simulink model "os_polyphase_synth_4096c"  (R2021a) (continued)
== system os_polyphase_synth_4096c/pfb_fir/pol1_in1_tap44 == (continued)
      | PCIN>>17-(D-A)*B-P-CARRYIN
      | PCIN>>17-(D-A)*BCIN
      | PCIN>>17-(D-A)*BCIN-C
      | PCIN>>17-(D-A)*BCIN-C-CARRYIN
      | PCIN>>17-(D-A)*BCIN-CARRYIN
      | PCIN>>17-(D-A)*BCIN-P
      | PCIN>>17-(D-A)*BCIN-P-CARRYIN
      | PCIN>>17-(D-A)-C
      | PCIN>>17-(D-A)-C-CARRYIN
      | PCIN>>17-(D-A)-CARRYIN
      | PCIN>>17-(D-A)-P
      | PCIN>>17-(D-A)-P-CARRYIN
      | PCIN>>17-(D-ACIN)
      | PCIN>>17-(D-ACIN)*(D-ACIN)
      | PCIN>>17-(D-ACIN)*(D-ACIN)-C
      | PCIN>>17-(D-ACIN)*(D-ACIN)-C-CARRYIN
      | PCIN>>17-(D-ACIN)*(D-ACIN)-CARRYIN
      | PCIN>>17-(D-ACIN)*(D-ACIN)-P
      | PCIN>>17-(D-ACIN)*(D-ACIN)-P-CARRYIN
      | PCIN>>17-(D-ACIN)*B
      | PCIN>>17-(D-ACIN)*B-C
      | PCIN>>17-(D-ACIN)*B-C-CARRYIN
      | PCIN>>17-(D-ACIN)*B-CARRYIN
      | PCIN>>17-(D-ACIN)*B-P
      | PCIN>>17-(D-ACIN)*B-P-CARRYIN
      | PCIN>>17-(D-ACIN)*BCIN
      | PCIN>>17-(D-ACIN)*BCIN-C
      | PCIN>>17-(D-ACIN)*BCIN-C-CARRYIN
      | PCIN>>17-(D-ACIN)*BCIN-CARRYIN
      | PCIN>>17-(D-ACIN)*BCIN-P
      | PCIN>>17-(D-ACIN)*BCIN-P-CARRYIN
      | PCIN>>17-(D-ACIN)-C
      | PCIN>>17-(D-ACIN)-C-CARRYIN
      | PCIN>>17-(D-ACIN)-CARRYIN
      | PCIN>>17-(D-ACIN)-P
      | PCIN>>17-(D-ACIN)-P-CARRYIN
      | PCIN>>17-(P)
      | PCIN>>17-(P+(A+D))
      | PCIN>>17-(P+(A+D)*(A+D))
      | PCIN>>17-(P+(A+D)*(A+D)+CARRYIN)
      | PCIN>>17-(P+(A+D)*B)
      | PCIN>>17-(P+(A+D)*B+CARRYIN)
      | PCIN>>17-(P+(A+D)*BCIN)
      | PCIN>>17-(P+(A+D)*BCIN+CARRYIN)
      | PCIN>>17-(P+(A+D)+CARRYIN)
      | PCIN>>17-(P+(ACIN+D))
      | PCIN>>17-(P+(ACIN+D)*(ACIN+D))
      | PCIN>>17-(P+(ACIN+D)*(ACIN+D)+CARRYIN)
      | PCIN>>17-(P+(ACIN+D)*B)
      | PCIN>>17-(P+(ACIN+D)*B+CARRYIN)
      | PCIN>>17-(P+(ACIN+D)*BCIN)
      | PCIN>>17-(P+(ACIN+D)*BCIN+CARRYIN)
      | PCIN>>17-(P+(ACIN+D)+CARRYIN)
      | PCIN>>17-(P+(D+A))
      | PCIN>>17-(P+(D+A)*(D+A))
      | PCIN>>17-(P+(D+A)*(D+A)+CARRYIN)
      | PCIN>>17-(P+(D+A)*B)
      | PCIN>>17-(P+(D+A)*B+CARRYIN)
      | PCIN>>17-(P+(D+A)*BCIN)
      | PCIN>>17-(P+(D+A)*BCIN+CARRYIN)
      | PCIN>>17-(P+(D+A)+CARRYIN)
      | PCIN>>17-(P+(D+ACIN))
      | PCIN>>17-(P+(D+ACIN)*(D+ACIN))
      | PCIN>>17-(P+(D+ACIN)*(D+ACIN)+CARRYIN)
      | PCIN>>17-(P+(D+ACIN)*B)
      | PCIN>>17-(P+(D+ACIN)*B+CARRYIN)
      | PCIN>>17-(P+(D+ACIN)*BCIN)
      | PCIN>>17-(P+(D+ACIN)*BCIN+CARRYIN)
      | PCIN>>17-(P+(D+ACIN)+CARRYIN)
      | PCIN>>17-(P+(D-A))
      | PCIN>>17-(P+(D-A)*(D-A))
      | PCIN>>17-(P+(D-A)*(D-A)+CARRYIN)
      | PCIN>>17-(P+(D-A)*B)
      | PCIN>>17-(P+(D-A)*B+CARRYIN)
      | PCIN>>17-(P+(D-A)*BCIN)
      | PCIN>>17-(P+(D-A)*BCIN+CARRYIN)
      | PCIN>>17-(P+(D-A)+CARRYIN)
      | PCIN>>17-(P+(D-ACIN))
      | PCIN>>17-(P+(D-ACIN)*(D-ACIN))
      | PCIN>>17-(P+(D-ACIN)*(D-ACIN)+CARRYIN)
      | PCIN>>17-(P+(D-ACIN)*B)
      | PCIN>>17-(P+(D-ACIN)*B+CARRYIN)
      | PCIN>>17-(P+(D-ACIN)*BCIN)
      | PCIN>>17-(P+(D-ACIN)*BCIN+CARRYIN)
      | PCIN>>17-(P+(D-ACIN)+CARRYIN)
      | PCIN>>17-(P+A)
      | PCIN>>17-(P+A*A)
      | PCIN>>17-(P+A*A+CARRYIN)
      | PCIN>>17-(P+A*B)
      | PCIN>>17-(P+A*B+CARRYIN)
      | PCIN>>17-(P+A*BCIN)
      | PCIN>>17-(P+A*BCIN+CARRYIN)
      | PCIN>>17-(P+A+CARRYIN)
      | PCIN>>17-(P+ACIN)
      | PCIN>>17-(P+ACIN*ACIN)
      | PCIN>>17-(P+ACIN*ACIN+CARRYIN)
      | PCIN>>17-(P+ACIN*B)
      | PCIN>>17-(P+ACIN*B+CARRYIN)
      | PCIN>>17-(P+ACIN*BCIN)
      | PCIN>>17-(P+ACIN*BCIN+CARRYIN)
      | PCIN>>17-(P+ACIN+CARRYIN)
      | PCIN>>17-(P+B*(A+D))
      | PCIN>>17-(P+B*(A+D)+CARRYIN)
      | PCIN>>17-(P+B*(ACIN+D))
      | PCIN>>17-(P+B*(ACIN+D)+CARRYIN)
      | PCIN>>17-(P+B*(D+A))
      | PCIN>>17-(P+B*(D+A)+CARRYIN)
      | PCIN>>17-(P+B*(D+ACIN))
      | PCIN>>17-(P+B*(D+ACIN)+CARRYIN)
      | PCIN>>17-(P+B*(D-A))
      | PCIN>>17-(P+B*(D-A)+CARRYIN)
      | PCIN>>17-(P+B*(D-ACIN))
      | PCIN>>17-(P+B*(D-ACIN)+CARRYIN)
      | PCIN>>17-(P+B*A)
      | PCIN>>17-(P+B*A+CARRYIN)
      | PCIN>>17-(P+B*ACIN)
      | PCIN>>17-(P+B*ACIN+CARRYIN)
      | PCIN>>17-(P+B*D)
      | PCIN>>17-(P+B*D+CARRYIN)
      | PCIN>>17-(P+BCIN*(A+D))
      | PCIN>>17-(P+BCIN*(A+D)+CARRYIN)
      | PCIN>>17-(P+BCIN*(ACIN+D))
      | PCIN>>17-(P+BCIN*(ACIN+D)+CARRYIN)
      | PCIN>>17-(P+BCIN*(D+A))
      | PCIN>>17-(P+BCIN*(D+A)+CARRYIN)
      | PCIN>>17-(P+BCIN*(D+ACIN))
      | PCIN>>17-(P+BCIN*(D+ACIN)+CARRYIN)
      | PCIN>>17-(P+BCIN*(D-A))
      | PCIN>>17-(P+BCIN*(D-A)+CARRYIN)
      | PCIN>>17-(P+BCIN*(D-ACIN))
      | PCIN>>17-(P+BCIN*(D-ACIN)+CARRYIN)
      | PCIN>>17-(P+BCIN*A)
      | PCIN>>17-(P+BCIN*A+CARRYIN)
      | PCIN>>17-(P+BCIN*ACIN)
      | PCIN>>17-(P+BCIN*ACIN+CARRYIN)
      | PCIN>>17-(P+BCIN*D)
      | PCIN>>17-(P+BCIN*D+CARRYIN)
      | PCIN>>17-(P+C)
      | PCIN>>17-(P+C+C)
      | PCIN>>17-(P+C+C+CARRYIN)
      | PCIN>>17-(P+C+CARRYIN)
      | PCIN>>17-(P+C+CONCAT)
      | PCIN>>17-(P+C+CONCAT+CARRYIN)
      | PCIN>>17-(P+C+P)
      | PCIN>>17-(P+C+P+CARRYIN)
      | PCIN>>17-(P+CARRYIN)
      | PCIN>>17-(P+CONCAT)
      | PCIN>>17-(P+CONCAT+C)
      | PCIN>>17-(P+CONCAT+C+CARRYIN)
      | PCIN>>17-(P+CONCAT+CARRYIN)
      | PCIN>>17-(P+D)
      | PCIN>>17-(P+D*B)
      | PCIN>>17-(P+D*B+CARRYIN)
      | PCIN>>17-(P+D*BCIN)
      | PCIN>>17-(P+D*BCIN+CARRYIN)
      | PCIN>>17-(P+D*D)
      | PCIN>>17-(P+D*D+CARRYIN)
      | PCIN>>17-(P+D+CARRYIN)
      | PCIN>>17-(P+P)
      | PCIN>>17-(P+P+C)
      | PCIN>>17-(P+P+C+CARRYIN)
      | PCIN>>17-(P+P+CARRYIN)
      | PCIN>>17-A
      | PCIN>>17-A*A
      | PCIN>>17-A*A-C
      | PCIN>>17-A*A-C-CARRYIN
      | PCIN>>17-A*A-CARRYIN
      | PCIN>>17-A*A-P
      | PCIN>>17-A*A-P-CARRYIN
      | PCIN>>17-A*B
      | PCIN>>17-A*B-C
      | PCIN>>17-A*B-C-CARRYIN
      | PCIN>>17-A*B-CARRYIN
      | PCIN>>17-A*B-P
      | PCIN>>17-A*B-P-CARRYIN
      | PCIN>>17-A*BCIN
      | PCIN>>17-A*BCIN-C
      | PCIN>>17-A*BCIN-C-CARRYIN
      | PCIN>>17-A*BCIN-CARRYIN
      | PCIN>>17-A*BCIN-P
      | PCIN>>17-A*BCIN-P-CARRYIN
      | PCIN>>17-A-C
      | PCIN>>17-A-C-CARRYIN
      | PCIN>>17-A-CARRYIN
      | PCIN>>17-A-P
      | PCIN>>17-A-P-CARRYIN
      | PCIN>>17-ACIN
      | PCIN>>17-ACIN*ACIN
      | PCIN>>17-ACIN*ACIN-C
      | PCIN>>17-ACIN*ACIN-C-CARRYIN
      | PCIN>>17-ACIN*ACIN-CARRYIN
      | PCIN>>17-ACIN*ACIN-P
      | PCIN>>17-ACIN*ACIN-P-CARRYIN
      | PCIN>>17-ACIN*B
      | PCIN>>17-ACIN*B-C
      | PCIN>>17-ACIN*B-C-CARRYIN
      | PCIN>>17-ACIN*B-CARRYIN
      | PCIN>>17-ACIN*B-P
      | PCIN>>17-ACIN*B-P-CARRYIN
      | PCIN>>17-ACIN*BCIN
      | PCIN>>17-ACIN*BCIN-C
      | PCIN>>17-ACIN*BCIN-C-CARRYIN
      | PCIN>>17-ACIN*BCIN-CARRYIN
      | PCIN>>17-ACIN*BCIN-P
      | PCIN>>17-ACIN*BCIN-P-CARRYIN
      | PCIN>>17-ACIN-C
      | PCIN>>17-ACIN-C-CARRYIN
      | PCIN>>17-ACIN-CARRYIN
      | PCIN>>17-ACIN-P
      | PCIN>>17-ACIN-P-CARRYIN
      | PCIN>>17-B*(A+D)
      | PCIN>>17-B*(A+D)-C
      | PCIN>>17-B*(A+D)-C-CARRYIN
      | PCIN>>17-B*(A+D)-CARRYIN
      | PCIN>>17-B*(A+D)-P
      | PCIN>>17-B*(A+D)-P-CARRYIN
      | PCIN>>17-B*(ACIN+D)
      | PCIN>>17-B*(ACIN+D)-C
      | PCIN>>17-B*(ACIN+D)-C-CARRYIN
      | PCIN>>17-B*(ACIN+D)-CARRYIN
      | PCIN>>17-B*(ACIN+D)-P
      | PCIN>>17-B*(ACIN+D)-P-CARRYIN
      | PCIN>>17-B*(D+A)
      | PCIN>>17-B*(D+A)-C
      | PCIN>>17-B*(D+A)-C-CARRYIN
      | PCIN>>17-B*(D+A)-CARRYIN
      | PCIN>>17-B*(D+A)-P
      | PCIN>>17-B*(D+A)-P-CARRYIN
      | PCIN>>17-B*(D+ACIN)
      | PCIN>>17-B*(D+ACIN)-C
      | PCIN>>17-B*(D+ACIN)-C-CARRYIN
      | PCIN>>17-B*(D+ACIN)-CARRYIN
      | PCIN>>17-B*(D+ACIN)-P
      | PCIN>>17-B*(D+ACIN)-P-CARRYIN
      | PCIN>>17-B*(D-A)
      | PCIN>>17-B*(D-A)-C
      | PCIN>>17-B*(D-A)-C-CARRYIN
      | PCIN>>17-B*(D-A)-CARRYIN
      | PCIN>>17-B*(D-A)-P
      | PCIN>>17-B*(D-A)-P-CARRYIN
      | PCIN>>17-B*(D-ACIN)
      | PCIN>>17-B*(D-ACIN)-C
      | PCIN>>17-B*(D-ACIN)-C-CARRYIN
      | PCIN>>17-B*(D-ACIN)-CARRYIN
      | PCIN>>17-B*(D-ACIN)-P
      | PCIN>>17-B*(D-ACIN)-P-CARRYIN
      | PCIN>>17-B*A
      | PCIN>>17-B*A-C
      | PCIN>>17-B*A-C-CARRYIN
      | PCIN>>17-B*A-CARRYIN
      | PCIN>>17-B*A-P
      | PCIN>>17-B*A-P-CARRYIN
      | PCIN>>17-B*ACIN
      | PCIN>>17-B*ACIN-C
      | PCIN>>17-B*ACIN-C-CARRYIN
      | PCIN>>17-B*ACIN-CARRYIN
      | PCIN>>17-B*ACIN-P
      | PCIN>>17-B*ACIN-P-CARRYIN
      | PCIN>>17-B*D
      | PCIN>>17-B*D-C
      | PCIN>>17-B*D-C-CARRYIN
      | PCIN>>17-B*D-CARRYIN
      | PCIN>>17-B*D-P
      | PCIN>>17-B*D-P-CARRYIN
      | PCIN>>17-BCIN*(A+D)
      | PCIN>>17-BCIN*(A+D)-C
      | PCIN>>17-BCIN*(A+D)-C-CARRYIN
      | PCIN>>17-BCIN*(A+D)-CARRYIN
      | PCIN>>17-BCIN*(A+D)-P
      | PCIN>>17-BCIN*(A+D)-P-CARRYIN
      | PCIN>>17-BCIN*(ACIN+D)
      | PCIN>>17-BCIN*(ACIN+D)-C
      | PCIN>>17-BCIN*(ACIN+D)-C-CARRYIN
      | PCIN>>17-BCIN*(ACIN+D)-CARRYIN
      | PCIN>>17-BCIN*(ACIN+D)-P
      | PCIN>>17-BCIN*(ACIN+D)-P-CARRYIN
      | PCIN>>17-BCIN*(D+A)
      | PCIN>>17-BCIN*(D+A)-C
      | PCIN>>17-BCIN*(D+A)-C-CARRYIN
      | PCIN>>17-BCIN*(D+A)-CARRYIN
      | PCIN>>17-BCIN*(D+A)-P
      | PCIN>>17-BCIN*(D+A)-P-CARRYIN
      | PCIN>>17-BCIN*(D+ACIN)
      | PCIN>>17-BCIN*(D+ACIN)-C
      | PCIN>>17-BCIN*(D+ACIN)-C-CARRYIN
      | PCIN>>17-BCIN*(D+ACIN)-CARRYIN
      | PCIN>>17-BCIN*(D+ACIN)-P
      | PCIN>>17-BCIN*(D+ACIN)-P-CARRYIN
      | PCIN>>17-BCIN*(D-A)
      | PCIN>>17-BCIN*(D-A)-C
      | PCIN>>17-BCIN*(D-A)-C-CARRYIN
      | PCIN>>17-BCIN*(D-A)-CARRYIN
      | PCIN>>17-BCIN*(D-A)-P
      | PCIN>>17-BCIN*(D-A)-P-CARRYIN
      | PCIN>>17-BCIN*(D-ACIN)
      | PCIN>>17-BCIN*(D-ACIN)-C
      | PCIN>>17-BCIN*(D-ACIN)-C-CARRYIN
      | PCIN>>17-BCIN*(D-ACIN)-CARRYIN
      | PCIN>>17-BCIN*(D-ACIN)-P
      | PCIN>>17-BCIN*(D-ACIN)-P-CARRYIN
      | PCIN>>17-BCIN*A
      | PCIN>>17-BCIN*A-C
      | PCIN>>17-BCIN*A-C-CARRYIN
      | PCIN>>17-BCIN*A-CARRYIN
      | PCIN>>17-BCIN*A-P
      | PCIN>>17-BCIN*A-P-CARRYIN
      | PCIN>>17-BCIN*ACIN
      | PCIN>>17-BCIN*ACIN-C
      | PCIN>>17-BCIN*ACIN-C-CARRYIN
      | PCIN>>17-BCIN*ACIN-CARRYIN
      | PCIN>>17-BCIN*ACIN-P
      | PCIN>>17-BCIN*ACIN-P-CARRYIN
      | PCIN>>17-BCIN*D
      | PCIN>>17-BCIN*D-C
      | PCIN>>17-BCIN*D-C-CARRYIN
      | PCIN>>17-BCIN*D-CARRYIN
      | PCIN>>17-BCIN*D-P
      | PCIN>>17-BCIN*D-P-CARRYIN
      | PCIN>>17-C
      | PCIN>>17-C-(A+D)
      | PCIN>>17-C-(A+D)*(A+D)
      | PCIN>>17-C-(A+D)*(A+D)-CARRYIN
      | PCIN>>17-C-(A+D)*B
      | PCIN>>17-C-(A+D)*B-CARRYIN
      | PCIN>>17-C-(A+D)*BCIN
      | PCIN>>17-C-(A+D)*BCIN-CARRYIN
      | PCIN>>17-C-(A+D)-CARRYIN
      | PCIN>>17-C-(ACIN+D)
      | PCIN>>17-C-(ACIN+D)*(ACIN+D)
      | PCIN>>17-C-(ACIN+D)*(ACIN+D)-CARRYIN
      | PCIN>>17-C-(ACIN+D)*B
      | PCIN>>17-C-(ACIN+D)*B-CARRYIN
      | PCIN>>17-C-(ACIN+D)*BCIN
      | PCIN>>17-C-(ACIN+D)*BCIN-CARRYIN
      | PCIN>>17-C-(ACIN+D)-CARRYIN
      | PCIN>>17-C-(D+A)
      | PCIN>>17-C-(D+A)*(D+A)
      | PCIN>>17-C-(D+A)*(D+A)-CARRYIN
      | PCIN>>17-C-(D+A)*B
      | PCIN>>17-C-(D+A)*B-CARRYIN
      | PCIN>>17-C-(D+A)*BCIN
      | PCIN>>17-C-(D+A)*BCIN-CARRYIN
      | PCIN>>17-C-(D+A)-CARRYIN
      | PCIN>>17-C-(D+ACIN)
      | PCIN>>17-C-(D+ACIN)*(D+ACIN)
      | PCIN>>17-C-(D+ACIN)*(D+ACIN)-CARRYIN
      | PCIN>>17-C-(D+ACIN)*B
      | PCIN>>17-C-(D+ACIN)*B-CARRYIN
      | PCIN>>17-C-(D+ACIN)*BCIN
      | PCIN>>17-C-(D+ACIN)*BCIN-CARRYIN
      | PCIN>>17-C-(D+ACIN)-CARRYIN
      | PCIN>>17-C-(D-A)
      | PCIN>>17-C-(D-A)*(D-A)
      | PCIN>>17-C-(D-A)*(D-A)-CARRYIN
      | PCIN>>17-C-(D-A)*B
      | PCIN>>17-C-(D-A)*B-CARRYIN
      | PCIN>>17-C-(D-A)*BCIN
      | PCIN>>17-C-(D-A)*BCIN-CARRYIN
      | PCIN>>17-C-(D-A)-CARRYIN
      | PCIN>>17-C-(D-ACIN)
      | PCIN>>17-C-(D-ACIN)*(D-ACIN)
      | PCIN>>17-C-(D-ACIN)*(D-ACIN)-CARRYIN
      | PCIN>>17-C-(D-ACIN)*B
      | PCIN>>17-C-(D-ACIN)*B-CARRYIN
      | PCIN>>17-C-(D-ACIN)*BCIN
      | PCIN>>17-C-(D-ACIN)*BCIN-CARRYIN
      | PCIN>>17-C-(D-ACIN)-CARRYIN
      | PCIN>>17-C-A
      | PCIN>>17-C-A*A
      | PCIN>>17-C-A*A-CARRYIN
      | PCIN>>17-C-A*B
      | PCIN>>17-C-A*B-CARRYIN
      | PCIN>>17-C-A*BCIN
      | PCIN>>17-C-A*BCIN-CARRYIN
      | PCIN>>17-C-A-CARRYIN
      | PCIN>>17-C-ACIN
      | PCIN>>17-C-ACIN*ACIN
      | PCIN>>17-C-ACIN*ACIN-CARRYIN
      | PCIN>>17-C-ACIN*B
      | PCIN>>17-C-ACIN*B-CARRYIN
      | PCIN>>17-C-ACIN*BCIN
      | PCIN>>17-C-ACIN*BCIN-CARRYIN
      | PCIN>>17-C-ACIN-CARRYIN
      | PCIN>>17-C-B*(A+D)
      | PCIN>>17-C-B*(A+D)-CARRYIN
      | PCIN>>17-C-B*(ACIN+D)
      | PCIN>>17-C-B*(ACIN+D)-CARRYIN
      | PCIN>>17-C-B*(D+A)
      | PCIN>>17-C-B*(D+A)-CARRYIN
      | PCIN>>17-C-B*(D+ACIN)
      | PCIN>>17-C-B*(D+ACIN)-CARRYIN
      | PCIN>>17-C-B*(D-A)
      | PCIN>>17-C-B*(D-A)-CARRYIN
      | PCIN>>17-C-B*(D-ACIN)
      | PCIN>>17-C-B*(D-ACIN)-CARRYIN
      | PCIN>>17-C-B*A
      | PCIN>>17-C-B*A-CARRYIN
      | PCIN>>17-C-B*ACIN
      | PCIN>>17-C-B*ACIN-CARRYIN
      | PCIN>>17-C-B*D
      | PCIN>>17-C-B*D-CARRYIN
      | PCIN>>17-C-BCIN*(A+D)
      | PCIN>>17-C-BCIN*(A+D)-CARRYIN
      | PCIN>>17-C-BCIN*(ACIN+D)
      | PCIN>>17-C-BCIN*(ACIN+D)-CARRYIN
      | PCIN>>17-C-BCIN*(D+A)
      | PCIN>>17-C-BCIN*(D+A)-CARRYIN
      | PCIN>>17-C-BCIN*(D+ACIN)
      | PCIN>>17-C-BCIN*(D+ACIN)-CARRYIN
      | PCIN>>17-C-BCIN*(D-A)
      | PCIN>>17-C-BCIN*(D-A)-CARRYIN
      | PCIN>>17-C-BCIN*(D-ACIN)
      | PCIN>>17-C-BCIN*(D-ACIN)-CARRYIN
      | PCIN>>17-C-BCIN*A
      | PCIN>>17-C-BCIN*A-CARRYIN
      | PCIN>>17-C-BCIN*ACIN
      | PCIN>>17-C-BCIN*ACIN-CARRYIN
      | PCIN>>17-C-BCIN*D
      | PCIN>>17-C-BCIN*D-CARRYIN
      | PCIN>>17-C-C
      | PCIN>>17-C-C-CARRYIN
      | PCIN>>17-C-C-CONCAT
      | PCIN>>17-C-C-CONCAT-CARRYIN
      | PCIN>>17-C-C-P
      | PCIN>>17-C-C-P-CARRYIN
      | PCIN>>17-C-CARRYIN
      | PCIN>>17-C-CONCAT
      | PCIN>>17-C-CONCAT-C
      | PCIN>>17-C-CONCAT-C-CARRYIN
      | PCIN>>17-C-CONCAT-CARRYIN
      | PCIN>>17-C-CONCAT-P
      | PCIN>>17-C-CONCAT-P-CARRYIN
      | PCIN>>17-C-D
      | PCIN>>17-C-D*B
      | PCIN>>17-C-D*B-CARRYIN
      | PCIN>>17-C-D*BCIN
      | PCIN>>17-C-D*BCIN-CARRYIN
      | PCIN>>17-C-D*D
      | PCIN>>17-C-D*D-CARRYIN
      | PCIN>>17-C-D-CARRYIN
      | PCIN>>17-C-P
      | PCIN>>17-C-P-C
      | PCIN>>17-C-P-C-CARRYIN
      | PCIN>>17-C-P-CARRYIN
      | PCIN>>17-C-P-CONCAT
      | PCIN>>17-C-P-CONCAT-CARRYIN
      | PCIN>>17-C-P-P
      | PCIN>>17-C-P-P-CARRYIN
      | PCIN>>17-CARRYIN
      | PCIN>>17-CONCAT
      | PCIN>>17-CONCAT-C
      | PCIN>>17-CONCAT-C-C
      | PCIN>>17-CONCAT-C-C-CARRYIN
      | PCIN>>17-CONCAT-C-CARRYIN
      | PCIN>>17-CONCAT-C-P
      | PCIN>>17-CONCAT-C-P-CARRYIN
      | PCIN>>17-CONCAT-CARRYIN
      | PCIN>>17-CONCAT-P
      | PCIN>>17-CONCAT-P-C
      | PCIN>>17-CONCAT-P-C-CARRYIN
      | PCIN>>17-CONCAT-P-CARRYIN
      | PCIN>>17-D
      | PCIN>>17-D*B
      | PCIN>>17-D*B-C
      | PCIN>>17-D*B-C-CARRYIN
      | PCIN>>17-D*B-CARRYIN
      | PCIN>>17-D*B-P
      | PCIN>>17-D*B-P-CARRYIN
      | PCIN>>17-D*BCIN
      | PCIN>>17-D*BCIN-C
      | PCIN>>17-D*BCIN-C-CARRYIN
      | PCIN>>17-D*BCIN-CARRYIN
      | PCIN>>17-D*BCIN-P
      | PCIN>>17-D*BCIN-P-CARRYIN
      | PCIN>>17-D*D
      | PCIN>>17-D*D-C
      | PCIN>>17-D*D-C-CARRYIN
      | PCIN>>17-D*D-CARRYIN
      | PCIN>>17-D*D-P
      | PCIN>>17-D*D-P-CARRYIN
      | PCIN>>17-D-C
      | PCIN>>17-D-C-CARRYIN
      | PCIN>>17-D-CARRYIN
      | PCIN>>17-D-P
      | PCIN>>17-D-P-CARRYIN
      | PCIN>>17-P
      | PCIN>>17-P-(A+D)
      | PCIN>>17-P-(A+D)*(A+D)
      | PCIN>>17-P-(A+D)*(A+D)-CARRYIN
      | PCIN>>17-P-(A+D)*B
      | PCIN>>17-P-(A+D)*B-CARRYIN
      | PCIN>>17-P-(A+D)*BCIN
      | PCIN>>17-P-(A+D)*BCIN-CARRYIN
      | PCIN>>17-P-(A+D)-CARRYIN
      | PCIN>>17-P-(ACIN+D)
      | PCIN>>17-P-(ACIN+D)*(ACIN+D)
      | PCIN>>17-P-(ACIN+D)*(ACIN+D)-CARRYIN
      | PCIN>>17-P-(ACIN+D)*B
      | PCIN>>17-P-(ACIN+D)*B-CARRYIN
      | PCIN>>17-P-(ACIN+D)*BCIN
      | PCIN>>17-P-(ACIN+D)*BCIN-CARRYIN
      | PCIN>>17-P-(ACIN+D)-CARRYIN
      | PCIN>>17-P-(D+A)
      | PCIN>>17-P-(D+A)*(D+A)
      | PCIN>>17-P-(D+A)*(D+A)-CARRYIN
      | PCIN>>17-P-(D+A)*B
      | PCIN>>17-P-(D+A)*B-CARRYIN
      | PCIN>>17-P-(D+A)*BCIN
      | PCIN>>17-P-(D+A)*BCIN-CARRYIN
      | PCIN>>17-P-(D+A)-CARRYIN
      | PCIN>>17-P-(D+ACIN)
      | PCIN>>17-P-(D+ACIN)*(D+ACIN)
      | PCIN>>17-P-(D+ACIN)*(D+ACIN)-CARRYIN
      | PCIN>>17-P-(D+ACIN)*B
      | PCIN>>17-P-(D+ACIN)*B-CARRYIN
      | PCIN>>17-P-(D+ACIN)*BCIN
      | PCIN>>17-P-(D+ACIN)*BCIN-CARRYIN
      | PCIN>>17-P-(D+ACIN)-CARRYIN
      | PCIN>>17-P-(D-A)
      | PCIN>>17-P-(D-A)*(D-A)
      | PCIN>>17-P-(D-A)*(D-A)-CARRYIN
      | PCIN>>17-P-(D-A)*B
      | PCIN>>17-P-(D-A)*B-CARRYIN
      | PCIN>>17-P-(D-A)*BCIN
      | PCIN>>17-P-(D-A)*BCIN-CARRYIN
      | PCIN>>17-P-(D-A)-CARRYIN
      | PCIN>>17-P-(D-ACIN)
      | PCIN>>17-P-(D-ACIN)*(D-ACIN)
      | PCIN>>17-P-(D-ACIN)*(D-ACIN)-CARRYIN
      | PCIN>>17-P-(D-ACIN)*B
      | PCIN>>17-P-(D-ACIN)*B-CARRYIN
      | PCIN>>17-P-(D-ACIN)*BCIN
      | PCIN>>17-P-(D-ACIN)*BCIN-CARRYIN
      | PCIN>>17-P-(D-ACIN)-CARRYIN
      | PCIN>>17-P-A
      | PCIN>>17-P-A*A
      | PCIN>>17-P-A*A-CARRYIN
      | PCIN>>17-P-A*B
      | PCIN>>17-P-A*B-CARRYIN
      | PCIN>>17-P-A*BCIN
      | PCIN>>17-P-A*BCIN-CARRYIN
      | PCIN>>17-P-A-CARRYIN
      | PCIN>>17-P-ACIN
      | PCIN>>17-P-ACIN*ACIN
      | PCIN>>17-P-ACIN*ACIN-CARRYIN
      | PCIN>>17-P-ACIN*B
      | PCIN>>17-P-ACIN*B-CARRYIN
      | PCIN>>17-P-ACIN*BCIN
      | PCIN>>17-P-ACIN*BCIN-CARRYIN
      | PCIN>>17-P-ACIN-CARRYIN
      | PCIN>>17-P-B*(A+D)
      | PCIN>>17-P-B*(A+D)-CARRYIN
      | PCIN>>17-P-B*(ACIN+D)
      | PCIN>>17-P-B*(ACIN+D)-CARRYIN
      | PCIN>>17-P-B*(D+A)
      | PCIN>>17-P-B*(D+A)-CARRYIN
      | PCIN>>17-P-B*(D+ACIN)
      | PCIN>>17-P-B*(D+ACIN)-CARRYIN
      | PCIN>>17-P-B*(D-A)
      | PCIN>>17-P-B*(D-A)-CARRYIN
      | PCIN>>17-P-B*(D-ACIN)
      | PCIN>>17-P-B*(D-ACIN)-CARRYIN
      | PCIN>>17-P-B*A
      | PCIN>>17-P-B*A-CARRYIN
      | PCIN>>17-P-B*ACIN
      | PCIN>>17-P-B*ACIN-CARRYIN
      | PCIN>>17-P-B*D
      | PCIN>>17-P-B*D-CARRYIN
      | PCIN>>17-P-BCIN*(A+D)
      | PCIN>>17-P-BCIN*(A+D)-CARRYIN
      | PCIN>>17-P-BCIN*(ACIN+D)
      | PCIN>>17-P-BCIN*(ACIN+D)-CARRYIN
      | PCIN>>17-P-BCIN*(D+A)
      | PCIN>>17-P-BCIN*(D+A)-CARRYIN
      | PCIN>>17-P-BCIN*(D+ACIN)
      | PCIN>>17-P-BCIN*(D+ACIN)-CARRYIN
      | PCIN>>17-P-BCIN*(D-A)
      | PCIN>>17-P-BCIN*(D-A)-CARRYIN
      | PCIN>>17-P-BCIN*(D-ACIN)
      | PCIN>>17-P-BCIN*(D-ACIN)-CARRYIN
      | PCIN>>17-P-BCIN*A
      | PCIN>>17-P-BCIN*A-CARRYIN
      | PCIN>>17-P-BCIN*ACIN
      | PCIN>>17-P-BCIN*ACIN-CARRYIN
      | PCIN>>17-P-BCIN*D
      | PCIN>>17-P-BCIN*D-CARRYIN
      | PCIN>>17-P-C
      | PCIN>>17-P-C-C
      | PCIN>>17-P-C-C-CARRYIN
      | PCIN>>17-P-C-CARRYIN
      | PCIN>>17-P-C-CONCAT
      | PCIN>>17-P-C-CONCAT-CARRYIN
      | PCIN>>17-P-C-P
      | PCIN>>17-P-C-P-CARRYIN
      | PCIN>>17-P-CARRYIN
      | PCIN>>17-P-CONCAT
      | PCIN>>17-P-CONCAT-C
      | PCIN>>17-P-CONCAT-C-CARRYIN
      | PCIN>>17-P-CONCAT-CARRYIN
      | PCIN>>17-P-D
      | PCIN>>17-P-D*B
      | PCIN>>17-P-D*B-CARRYIN
      | PCIN>>17-P-D*BCIN
      | PCIN>>17-P-D*BCIN-CARRYIN
      | PCIN>>17-P-D*D
      | PCIN>>17-P-D*D-CARRYIN
      | PCIN>>17-P-D-CARRYIN
      | PCIN>>17-P-P
      | PCIN>>17-P-P-C
      | PCIN>>17-P-P-C-CARRYIN
      | PCIN>>17-P-P-CARRYIN
      | RNDMACC((A+D)*(A+D)+C+P)
      | RNDMACC((A+D)*(A+D)+P)
      | RNDMACC((A+D)*(A+D)+P+C)
      | RNDMACC((A+D)*(A+D)+P+P)
      | RNDMACC((A+D)*B+C+P)
      | RNDMACC((A+D)*B+P)
      | RNDMACC((A+D)*B+P+C)
      | RNDMACC((A+D)*B+P+P)
      | RNDMACC((A+D)*BCIN+C+P)
      | RNDMACC((A+D)*BCIN+P)
      | RNDMACC((A+D)*BCIN+P+C)
      | RNDMACC((A+D)*BCIN+P+P)
      | RNDMACC((ACIN+D)*(ACIN+D)+C+P)
      | RNDMACC((ACIN+D)*(ACIN+D)+P)
      | RNDMACC((ACIN+D)*(ACIN+D)+P+C)
      | RNDMACC((ACIN+D)*(ACIN+D)+P+P)
      | RNDMACC((ACIN+D)*B+C+P)
      | RNDMACC((ACIN+D)*B+P)
      | RNDMACC((ACIN+D)*B+P+C)
      | RNDMACC((ACIN+D)*B+P+P)
      | RNDMACC((ACIN+D)*BCIN+C+P)
      | RNDMACC((ACIN+D)*BCIN+P)
      | RNDMACC((ACIN+D)*BCIN+P+C)
      | RNDMACC((ACIN+D)*BCIN+P+P)
      | RNDMACC((D+A)*(D+A)+C+P)
      | RNDMACC((D+A)*(D+A)+P)
      | RNDMACC((D+A)*(D+A)+P+C)
      | RNDMACC((D+A)*(D+A)+P+P)
      | RNDMACC((D+A)*B+C+P)
      | RNDMACC((D+A)*B+P)
      | RNDMACC((D+A)*B+P+C)
      | RNDMACC((D+A)*B+P+P)
      | RNDMACC((D+A)*BCIN+C+P)
      | RNDMACC((D+A)*BCIN+P)
      | RNDMACC((D+A)*BCIN+P+C)
      | RNDMACC((D+A)*BCIN+P+P)
      | RNDMACC((D+ACIN)*(D+ACIN)+C+P)
      | RNDMACC((D+ACIN)*(D+ACIN)+P)
      | RNDMACC((D+ACIN)*(D+ACIN)+P+C)
      | RNDMACC((D+ACIN)*(D+ACIN)+P+P)
      | RNDMACC((D+ACIN)*B+C+P)
      | RNDMACC((D+ACIN)*B+P)
      | RNDMACC((D+ACIN)*B+P+C)
      | RNDMACC((D+ACIN)*B+P+P)
      | RNDMACC((D+ACIN)*BCIN+C+P)
      | RNDMACC((D+ACIN)*BCIN+P)
      | RNDMACC((D+ACIN)*BCIN+P+C)
      | RNDMACC((D+ACIN)*BCIN+P+P)
      | RNDMACC((D-A)*(D-A)+C+P)
      | RNDMACC((D-A)*(D-A)+P)
      | RNDMACC((D-A)*(D-A)+P+C)
      | RNDMACC((D-A)*(D-A)+P+P)
      | RNDMACC((D-A)*B+C+P)
      | RNDMACC((D-A)*B+P)
      | RNDMACC((D-A)*B+P+C)
      | RNDMACC((D-A)*B+P+P)
      | RNDMACC((D-A)*BCIN+C+P)
      | RNDMACC((D-A)*BCIN+P)
      | RNDMACC((D-A)*BCIN+P+C)
      | RNDMACC((D-A)*BCIN+P+P)
      | RNDMACC((D-ACIN)*(D-ACIN)+C+P)
      | RNDMACC((D-ACIN)*(D-ACIN)+P)
      | RNDMACC((D-ACIN)*(D-ACIN)+P+C)
      | RNDMACC((D-ACIN)*(D-ACIN)+P+P)
      | RNDMACC((D-ACIN)*B+C+P)
      | RNDMACC((D-ACIN)*B+P)
      | RNDMACC((D-ACIN)*B+P+C)
      | RNDMACC((D-ACIN)*B+P+P)
      | RNDMACC((D-ACIN)*BCIN+C+P)
      | RNDMACC((D-ACIN)*BCIN+P)
      | RNDMACC((D-ACIN)*BCIN+P+C)
      | RNDMACC((D-ACIN)*BCIN+P+P)
      | RNDMACC(A*A+C+P)
      | RNDMACC(A*A+P)
      | RNDMACC(A*A+P+C)
      | RNDMACC(A*A+P+P)
      | RNDMACC(A*B+C+P)
      | RNDMACC(A*B+P)
      | RNDMACC(A*B+P+C)
      | RNDMACC(A*B+P+P)
      | RNDMACC(A*BCIN+C+P)
      | RNDMACC(A*BCIN+P)
      | RNDMACC(A*BCIN+P+C)
      | RNDMACC(A*BCIN+P+P)
      | RNDMACC(ACIN*ACIN+C+P)
      | RNDMACC(ACIN*ACIN+P)
      | RNDMACC(ACIN*ACIN+P+C)
      | RNDMACC(ACIN*ACIN+P+P)
      | RNDMACC(ACIN*B+C+P)
      | RNDMACC(ACIN*B+P)
      | RNDMACC(ACIN*B+P+C)
      | RNDMACC(ACIN*B+P+P)
      | RNDMACC(ACIN*BCIN+C+P)
      | RNDMACC(ACIN*BCIN+P)
      | RNDMACC(ACIN*BCIN+P+C)
      | RNDMACC(ACIN*BCIN+P+P)
      | RNDMACC(B*(A+D)+C+P)
      | RNDMACC(B*(A+D)+P)
      | RNDMACC(B*(A+D)+P+C)
      | RNDMACC(B*(A+D)+P+P)
      | RNDMACC(B*(ACIN+D)+C+P)
      | RNDMACC(B*(ACIN+D)+P)
      | RNDMACC(B*(ACIN+D)+P+C)
      | RNDMACC(B*(ACIN+D)+P+P)
      | RNDMACC(B*(D+A)+C+P)
      | RNDMACC(B*(D+A)+P)
      | RNDMACC(B*(D+A)+P+C)
      | RNDMACC(B*(D+A)+P+P)
      | RNDMACC(B*(D+ACIN)+C+P)
      | RNDMACC(B*(D+ACIN)+P)
      | RNDMACC(B*(D+ACIN)+P+C)
      | RNDMACC(B*(D+ACIN)+P+P)
      | RNDMACC(B*(D-A)+C+P)
      | RNDMACC(B*(D-A)+P)
      | RNDMACC(B*(D-A)+P+C)
      | RNDMACC(B*(D-A)+P+P)
      | RNDMACC(B*(D-ACIN)+C+P)
      | RNDMACC(B*(D-ACIN)+P)
      | RNDMACC(B*(D-ACIN)+P+C)
      | RNDMACC(B*(D-ACIN)+P+P)
      | RNDMACC(B*A+C+P)
      | RNDMACC(B*A+P)
      | RNDMACC(B*A+P+C)
      | RNDMACC(B*A+P+P)
      | RNDMACC(B*ACIN+C+P)
      | RNDMACC(B*ACIN+P)
      | RNDMACC(B*ACIN+P+C)
      | RNDMACC(B*ACIN+P+P)
      | RNDMACC(B*D+C+P)
      | RNDMACC(B*D+P)
      | RNDMACC(B*D+P+C)
      | RNDMACC(B*D+P+P)
      | RNDMACC(BCIN*(A+D)+C+P)
      | RNDMACC(BCIN*(A+D)+P)
      | RNDMACC(BCIN*(A+D)+P+C)
      | RNDMACC(BCIN*(A+D)+P+P)
      | RNDMACC(BCIN*(ACIN+D)+C+P)
      | RNDMACC(BCIN*(ACIN+D)+P)
      | RNDMACC(BCIN*(ACIN+D)+P+C)
      | RNDMACC(BCIN*(ACIN+D)+P+P)
      | RNDMACC(BCIN*(D+A)+C+P)
      | RNDMACC(BCIN*(D+A)+P)
      | RNDMACC(BCIN*(D+A)+P+C)
      | RNDMACC(BCIN*(D+A)+P+P)
      | RNDMACC(BCIN*(D+ACIN)+C+P)
      | RNDMACC(BCIN*(D+ACIN)+P)
      | RNDMACC(BCIN*(D+ACIN)+P+C)
      | RNDMACC(BCIN*(D+ACIN)+P+P)
      | RNDMACC(BCIN*(D-A)+C+P)
      | RNDMACC(BCIN*(D-A)+P)
      | RNDMACC(BCIN*(D-A)+P+C)
      | RNDMACC(BCIN*(D-A)+P+P)
      | RNDMACC(BCIN*(D-ACIN)+C+P)
      | RNDMACC(BCIN*(D-ACIN)+P)
      | RNDMACC(BCIN*(D-ACIN)+P+C)
      | RNDMACC(BCIN*(D-ACIN)+P+P)
      | RNDMACC(BCIN*A+C+P)
      | RNDMACC(BCIN*A+P)
      | RNDMACC(BCIN*A+P+C)
      | RNDMACC(BCIN*A+P+P)
      | RNDMACC(BCIN*ACIN+C+P)
      | RNDMACC(BCIN*ACIN+P)
      | RNDMACC(BCIN*ACIN+P+C)
      | RNDMACC(BCIN*ACIN+P+P)
      | RNDMACC(BCIN*D+C+P)
      | RNDMACC(BCIN*D+P)
      | RNDMACC(BCIN*D+P+C)
      | RNDMACC(BCIN*D+P+P)
      | RNDMACC(D*B+C+P)
      | RNDMACC(D*B+P)
      | RNDMACC(D*B+P+C)
      | RNDMACC(D*B+P+P)
      | RNDMACC(D*BCIN+C+P)
      | RNDMACC(D*BCIN+P)
      | RNDMACC(D*BCIN+P+C)
      | RNDMACC(D*BCIN+P+P)
      | RNDMACC(D*D+C+P)
      | RNDMACC(D*D+P)
      | RNDMACC(D*D+P+C)
      | RNDMACC(D*D+P+P)
      | RNDSIMPLE((A+D))
      | RNDSIMPLE((A+D)*(A+D))
      | RNDSIMPLE((A+D)*(A+D)+C)
      | RNDSIMPLE((A+D)*(A+D)+C+CARRYIN)
      | RNDSIMPLE((A+D)*(A+D)+CARRYIN)
      | RNDSIMPLE((A+D)*(A+D)+P)
      | RNDSIMPLE((A+D)*(A+D)+P+CARRYIN)
      | RNDSIMPLE((A+D)*(A+D)+P>>17)
      | RNDSIMPLE((A+D)*(A+D)+P>>17+CARRYIN)
      | RNDSIMPLE((A+D)*(A+D)+PCIN)
      | RNDSIMPLE((A+D)*(A+D)+PCIN+CARRYIN)
      | RNDSIMPLE((A+D)*(A+D)+PCIN>>17)
      | RNDSIMPLE((A+D)*(A+D)+PCIN>>17+CARRYIN)
      | RNDSIMPLE((A+D)*B)
      | RNDSIMPLE((A+D)*B+C)
      | RNDSIMPLE((A+D)*B+C+CARRYIN)
      | RNDSIMPLE((A+D)*B+CARRYIN)
      | RNDSIMPLE((A+D)*B+P)
      | RNDSIMPLE((A+D)*B+P+CARRYIN)
      | RNDSIMPLE((A+D)*B+P>>17)
      | RNDSIMPLE((A+D)*B+P>>17+CARRYIN)
      | RNDSIMPLE((A+D)*B+PCIN)
      | RNDSIMPLE((A+D)*B+PCIN+CARRYIN)
      | RNDSIMPLE((A+D)*B+PCIN>>17)
      | RNDSIMPLE((A+D)*B+PCIN>>17+CARRYIN)
      | RNDSIMPLE((A+D)*BCIN)
      | RNDSIMPLE((A+D)*BCIN+C)
      | RNDSIMPLE((A+D)*BCIN+C+CARRYIN)
      | RNDSIMPLE((A+D)*BCIN+CARRYIN)
      | RNDSIMPLE((A+D)*BCIN+P)
      | RNDSIMPLE((A+D)*BCIN+P+CARRYIN)
      | RNDSIMPLE((A+D)*BCIN+P>>17)
      | RNDSIMPLE((A+D)*BCIN+P>>17+CARRYIN)
      | RNDSIMPLE((A+D)*BCIN+PCIN)
      | RNDSIMPLE((A+D)*BCIN+PCIN+CARRYIN)
      | RNDSIMPLE((A+D)*BCIN+PCIN>>17)
      | RNDSIMPLE((A+D)*BCIN+PCIN>>17+CARRYIN)
      | RNDSIMPLE((A+D)+C)
      | RNDSIMPLE((A+D)+C+CARRYIN)
      | RNDSIMPLE((A+D)+CARRYIN)
      | RNDSIMPLE((A+D)+P)
      | RNDSIMPLE((A+D)+P+CARRYIN)
      | RNDSIMPLE((A+D)+P>>17)
      | RNDSIMPLE((A+D)+P>>17+CARRYIN)
      | RNDSIMPLE((A+D)+PCIN)
      | RNDSIMPLE((A+D)+PCIN+CARRYIN)
      | RNDSIMPLE((A+D)+PCIN>>17)
      | RNDSIMPLE((A+D)+PCIN>>17+CARRYIN)
      | RNDSIMPLE((ACIN+D))
      | RNDSIMPLE((ACIN+D)*(ACIN+D))
      | RNDSIMPLE((ACIN+D)*(ACIN+D)+C)
      | RNDSIMPLE((ACIN+D)*(ACIN+D)+C+CARRYIN)
      | RNDSIMPLE((ACIN+D)*(ACIN+D)+CARRYIN)
      | RNDSIMPLE((ACIN+D)*(ACIN+D)+P)
      | RNDSIMPLE((ACIN+D)*(ACIN+D)+P+CARRYIN)
      | RNDSIMPLE((ACIN+D)*(ACIN+D)+P>>17)
      | RNDSIMPLE((ACIN+D)*(ACIN+D)+P>>17+CARRYIN)
      | RNDSIMPLE((ACIN+D)*(ACIN+D)+PCIN)
      | RNDSIMPLE((ACIN+D)*(ACIN+D)+PCIN+CARRYIN)
      | RNDSIMPLE((ACIN+D)*(ACIN+D)+PCIN>>17)
      | RNDSIMPLE((ACIN+D)*(ACIN+D)+PCIN>>17+CARRYIN)
      | RNDSIMPLE((ACIN+D)*B)
      | RNDSIMPLE((ACIN+D)*B+C)
      | RNDSIMPLE((ACIN+D)*B+C+CARRYIN)
      | RNDSIMPLE((ACIN+D)*B+CARRYIN)
      | RNDSIMPLE((ACIN+D)*B+P)
      | RNDSIMPLE((ACIN+D)*B+P+CARRYIN)
      | RNDSIMPLE((ACIN+D)*B+P>>17)
      | RNDSIMPLE((ACIN+D)*B+P>>17+CARRYIN)
      | RNDSIMPLE((ACIN+D)*B+PCIN)
      | RNDSIMPLE((ACIN+D)*B+PCIN+CARRYIN)
      | RNDSIMPLE((ACIN+D)*B+PCIN>>17)
      | RNDSIMPLE((ACIN+D)*B+PCIN>>17+CARRYIN)
      | RNDSIMPLE((ACIN+D)*BCIN)
      | RNDSIMPLE((ACIN+D)*BCIN+C)
      | RNDSIMPLE((ACIN+D)*BCIN+C+CARRYIN)
      | RNDSIMPLE((ACIN+D)*BCIN+CARRYIN)
      | RNDSIMPLE((ACIN+D)*BCIN+P)
      | RNDSIMPLE((ACIN+D)*BCIN+P+CARRYIN)
      | RNDSIMPLE((ACIN+D)*BCIN+P>>17)
      | RNDSIMPLE((ACIN+D)*BCIN+P>>17+CARRYIN)
      | RNDSIMPLE((ACIN+D)*BCIN+PCIN)
      | RNDSIMPLE((ACIN+D)*BCIN+PCIN+CARRYIN)
      | RNDSIMPLE((ACIN+D)*BCIN+PCIN>>17)
      | RNDSIMPLE((ACIN+D)*BCIN+PCIN>>17+CARRYIN)
      | RNDSIMPLE((ACIN+D)+C)
      | RNDSIMPLE((ACIN+D)+C+CARRYIN)
      | RNDSIMPLE((ACIN+D)+CARRYIN)
      | RNDSIMPLE((ACIN+D)+P)
      | RNDSIMPLE((ACIN+D)+P+CARRYIN)
      | RNDSIMPLE((ACIN+D)+P>>17)
      | RNDSIMPLE((ACIN+D)+P>>17+CARRYIN)
      | RNDSIMPLE((ACIN+D)+PCIN)
      | RNDSIMPLE((ACIN+D)+PCIN+CARRYIN)
      | RNDSIMPLE((ACIN+D)+PCIN>>17)
      | RNDSIMPLE((ACIN+D)+PCIN>>17+CARRYIN)
      | RNDSIMPLE((D+A))
      | RNDSIMPLE((D+A)*(D+A))
      | RNDSIMPLE((D+A)*(D+A)+C)
      | RNDSIMPLE((D+A)*(D+A)+C+CARRYIN)
      | RNDSIMPLE((D+A)*(D+A)+CARRYIN)
      | RNDSIMPLE((D+A)*(D+A)+P)
      | RNDSIMPLE((D+A)*(D+A)+P+CARRYIN)
      | RNDSIMPLE((D+A)*(D+A)+P>>17)
      | RNDSIMPLE((D+A)*(D+A)+P>>17+CARRYIN)
      | RNDSIMPLE((D+A)*(D+A)+PCIN)
      | RNDSIMPLE((D+A)*(D+A)+PCIN+CARRYIN)
      | RNDSIMPLE((D+A)*(D+A)+PCIN>>17)
      | RNDSIMPLE((D+A)*(D+A)+PCIN>>17+CARRYIN)
      | RNDSIMPLE((D+A)*B)
      | RNDSIMPLE((D+A)*B+C)
      | RNDSIMPLE((D+A)*B+C+CARRYIN)
      | RNDSIMPLE((D+A)*B+CARRYIN)
      | RNDSIMPLE((D+A)*B+P)
      | RNDSIMPLE((D+A)*B+P+CARRYIN)
      | RNDSIMPLE((D+A)*B+P>>17)
      | RNDSIMPLE((D+A)*B+P>>17+CARRYIN)
      | RNDSIMPLE((D+A)*B+PCIN)
      | RNDSIMPLE((D+A)*B+PCIN+CARRYIN)
      | RNDSIMPLE((D+A)*B+PCIN>>17)
      | RNDSIMPLE((D+A)*B+PCIN>>17+CARRYIN)
      | RNDSIMPLE((D+A)*BCIN)
      | RNDSIMPLE((D+A)*BCIN+C)
      | RNDSIMPLE((D+A)*BCIN+C+CARRYIN)
      | RNDSIMPLE((D+A)*BCIN+CARRYIN)
      | RNDSIMPLE((D+A)*BCIN+P)
      | RNDSIMPLE((D+A)*BCIN+P+CARRYIN)
      | RNDSIMPLE((D+A)*BCIN+P>>17)
      | RNDSIMPLE((D+A)*BCIN+P>>17+CARRYIN)
      | RNDSIMPLE((D+A)*BCIN+PCIN)
      | RNDSIMPLE((D+A)*BCIN+PCIN+CARRYIN)
      | RNDSIMPLE((D+A)*BCIN+PCIN>>17)
      | RNDSIMPLE((D+A)*BCIN+PCIN>>17+CARRYIN)
      | RNDSIMPLE((D+A)+C)
      | RNDSIMPLE((D+A)+C+CARRYIN)
      | RNDSIMPLE((D+A)+CARRYIN)
      | RNDSIMPLE((D+A)+P)
      | RNDSIMPLE((D+A)+P+CARRYIN)
      | RNDSIMPLE((D+A)+P>>17)
      | RNDSIMPLE((D+A)+P>>17+CARRYIN)
      | RNDSIMPLE((D+A)+PCIN)
      | RNDSIMPLE((D+A)+PCIN+CARRYIN)
      | RNDSIMPLE((D+A)+PCIN>>17)
      | RNDSIMPLE((D+A)+PCIN>>17+CARRYIN)
      | RNDSIMPLE((D+ACIN))
      | RNDSIMPLE((D+ACIN)*(D+ACIN))
      | RNDSIMPLE((D+ACIN)*(D+ACIN)+C)
      | RNDSIMPLE((D+ACIN)*(D+ACIN)+C+CARRYIN)
      | RNDSIMPLE((D+ACIN)*(D+ACIN)+CARRYIN)
      | RNDSIMPLE((D+ACIN)*(D+ACIN)+P)
      | RNDSIMPLE((D+ACIN)*(D+ACIN)+P+CARRYIN)
      | RNDSIMPLE((D+ACIN)*(D+ACIN)+P>>17)
      | RNDSIMPLE((D+ACIN)*(D+ACIN)+P>>17+CARRYIN)
      | RNDSIMPLE((D+ACIN)*(D+ACIN)+PCIN)
      | RNDSIMPLE((D+ACIN)*(D+ACIN)+PCIN+CARRYIN)
      | RNDSIMPLE((D+ACIN)*(D+ACIN)+PCIN>>17)
      | RNDSIMPLE((D+ACIN)*(D+ACIN)+PCIN>>17+CARRYIN)
      | RNDSIMPLE((D+ACIN)*B)
      | RNDSIMPLE((D+ACIN)*B+C)
      | RNDSIMPLE((D+ACIN)*B+C+CARRYIN)
      | RNDSIMPLE((D+ACIN)*B+CARRYIN)
      | RNDSIMPLE((D+ACIN)*B+P)
      | RNDSIMPLE((D+ACIN)*B+P+CARRYIN)
      | RNDSIMPLE((D+ACIN)*B+P>>17)
      | RNDSIMPLE((D+ACIN)*B+P>>17+CARRYIN)
      | RNDSIMPLE((D+ACIN)*B+PCIN)
      | RNDSIMPLE((D+ACIN)*B+PCIN+CARRYIN)
      | RNDSIMPLE((D+ACIN)*B+PCIN>>17)
      | RNDSIMPLE((D+ACIN)*B+PCIN>>17+CARRYIN)
      | RNDSIMPLE((D+ACIN)*BCIN)
      | RNDSIMPLE((D+ACIN)*BCIN+C)
      | RNDSIMPLE((D+ACIN)*BCIN+C+CARRYIN)
      | RNDSIMPLE((D+ACIN)*BCIN+CARRYIN)
      | RNDSIMPLE((D+ACIN)*BCIN+P)
      | RNDSIMPLE((D+ACIN)*BCIN+P+CARRYIN)
      | RNDSIMPLE((D+ACIN)*BCIN+P>>17)
      | RNDSIMPLE((D+ACIN)*BCIN+P>>17+CARRYIN)
      | RNDSIMPLE((D+ACIN)*BCIN+PCIN)
      | RNDSIMPLE((D+ACIN)*BCIN+PCIN+CARRYIN)
      | RNDSIMPLE((D+ACIN)*BCIN+PCIN>>17)
      | RNDSIMPLE((D+ACIN)*BCIN+PCIN>>17+CARRYIN)
      | RNDSIMPLE((D+ACIN)+C)
      | RNDSIMPLE((D+ACIN)+C+CARRYIN)
      | RNDSIMPLE((D+ACIN)+CARRYIN)
      | RNDSIMPLE((D+ACIN)+P)
      | RNDSIMPLE((D+ACIN)+P+CARRYIN)
      | RNDSIMPLE((D+ACIN)+P>>17)
      | RNDSIMPLE((D+ACIN)+P>>17+CARRYIN)
      | RNDSIMPLE((D+ACIN)+PCIN)
      | RNDSIMPLE((D+ACIN)+PCIN+CARRYIN)
      | RNDSIMPLE((D+ACIN)+PCIN>>17)
      | RNDSIMPLE((D+ACIN)+PCIN>>17+CARRYIN)
      | RNDSIMPLE((D-A))
      | RNDSIMPLE((D-A)*(D-A))
      | RNDSIMPLE((D-A)*(D-A)+C)
      | RNDSIMPLE((D-A)*(D-A)+C+CARRYIN)
      | RNDSIMPLE((D-A)*(D-A)+CARRYIN)
      | RNDSIMPLE((D-A)*(D-A)+P)
      | RNDSIMPLE((D-A)*(D-A)+P+CARRYIN)
      | RNDSIMPLE((D-A)*(D-A)+P>>17)
      | RNDSIMPLE((D-A)*(D-A)+P>>17+CARRYIN)
      | RNDSIMPLE((D-A)*(D-A)+PCIN)
      | RNDSIMPLE((D-A)*(D-A)+PCIN+CARRYIN)
      | RNDSIMPLE((D-A)*(D-A)+PCIN>>17)
      | RNDSIMPLE((D-A)*(D-A)+PCIN>>17+CARRYIN)
      | RNDSIMPLE((D-A)*B)
      | RNDSIMPLE((D-A)*B+C)
      | RNDSIMPLE((D-A)*B+C+CARRYIN)
      | RNDSIMPLE((D-A)*B+CARRYIN)
      | RNDSIMPLE((D-A)*B+P)
      | RNDSIMPLE((D-A)*B+P+CARRYIN)
      | RNDSIMPLE((D-A)*B+P>>17)
      | RNDSIMPLE((D-A)*B+P>>17+CARRYIN)
      | RNDSIMPLE((D-A)*B+PCIN)
      | RNDSIMPLE((D-A)*B+PCIN+CARRYIN)
      | RNDSIMPLE((D-A)*B+PCIN>>17)
      | RNDSIMPLE((D-A)*B+PCIN>>17+CARRYIN)
      | RNDSIMPLE((D-A)*BCIN)
      | RNDSIMPLE((D-A)*BCIN+C)
      | RNDSIMPLE((D-A)*BCIN+C+CARRYIN)
      | RNDSIMPLE((D-A)*BCIN+CARRYIN)
      | RNDSIMPLE((D-A)*BCIN+P)
      | RNDSIMPLE((D-A)*BCIN+P+CARRYIN)
      | RNDSIMPLE((D-A)*BCIN+P>>17)
      | RNDSIMPLE((D-A)*BCIN+P>>17+CARRYIN)
      | RNDSIMPLE((D-A)*BCIN+PCIN)
      | RNDSIMPLE((D-A)*BCIN+PCIN+CARRYIN)
      | RNDSIMPLE((D-A)*BCIN+PCIN>>17)
      | RNDSIMPLE((D-A)*BCIN+PCIN>>17+CARRYIN)
      | RNDSIMPLE((D-A)+C)
      | RNDSIMPLE((D-A)+C+CARRYIN)
      | RNDSIMPLE((D-A)+CARRYIN)
      | RNDSIMPLE((D-A)+P)
      | RNDSIMPLE((D-A)+P+CARRYIN)
      | RNDSIMPLE((D-A)+P>>17)
      | RNDSIMPLE((D-A)+P>>17+CARRYIN)
      | RNDSIMPLE((D-A)+PCIN)
      | RNDSIMPLE((D-A)+PCIN+CARRYIN)
      | RNDSIMPLE((D-A)+PCIN>>17)
      | RNDSIMPLE((D-A)+PCIN>>17+CARRYIN)
      | RNDSIMPLE((D-ACIN))
      | RNDSIMPLE((D-ACIN)*(D-ACIN))
      | RNDSIMPLE((D-ACIN)*(D-ACIN)+C)
      | RNDSIMPLE((D-ACIN)*(D-ACIN)+C+CARRYIN)
      | RNDSIMPLE((D-ACIN)*(D-ACIN)+CARRYIN)
      | RNDSIMPLE((D-ACIN)*(D-ACIN)+P)
      | RNDSIMPLE((D-ACIN)*(D-ACIN)+P+CARRYIN)
      | RNDSIMPLE((D-ACIN)*(D-ACIN)+P>>17)
      | RNDSIMPLE((D-ACIN)*(D-ACIN)+P>>17+CARRYIN)
      | RNDSIMPLE((D-ACIN)*(D-ACIN)+PCIN)
      | RNDSIMPLE((D-ACIN)*(D-ACIN)+PCIN+CARRYIN)
      | RNDSIMPLE((D-ACIN)*(D-ACIN)+PCIN>>17)
      | RNDSIMPLE((D-ACIN)*(D-ACIN)+PCIN>>17+CARRYIN)
      | RNDSIMPLE((D-ACIN)*B)
      | RNDSIMPLE((D-ACIN)*B+C)
      | RNDSIMPLE((D-ACIN)*B+C+CARRYIN)
      | RNDSIMPLE((D-ACIN)*B+CARRYIN)
      | RNDSIMPLE((D-ACIN)*B+P)
      | RNDSIMPLE((D-ACIN)*B+P+CARRYIN)
      | RNDSIMPLE((D-ACIN)*B+P>>17)
      | RNDSIMPLE((D-ACIN)*B+P>>17+CARRYIN)
      | RNDSIMPLE((D-ACIN)*B+PCIN)
      | RNDSIMPLE((D-ACIN)*B+PCIN+CARRYIN)
      | RNDSIMPLE((D-ACIN)*B+PCIN>>17)
      | RNDSIMPLE((D-ACIN)*B+PCIN>>17+CARRYIN)
      | RNDSIMPLE((D-ACIN)*BCIN)
      | RNDSIMPLE((D-ACIN)*BCIN+C)
      | RNDSIMPLE((D-ACIN)*BCIN+C+CARRYIN)
      | RNDSIMPLE((D-ACIN)*BCIN+CARRYIN)
      | RNDSIMPLE((D-ACIN)*BCIN+P)
      | RNDSIMPLE((D-ACIN)*BCIN+P+CARRYIN)
      | RNDSIMPLE((D-ACIN)*BCIN+P>>17)
      | RNDSIMPLE((D-ACIN)*BCIN+P>>17+CARRYIN)
      | RNDSIMPLE((D-ACIN)*BCIN+PCIN)
      | RNDSIMPLE((D-ACIN)*BCIN+PCIN+CARRYIN)
      | RNDSIMPLE((D-ACIN)*BCIN+PCIN>>17)
      | RNDSIMPLE((D-ACIN)*BCIN+PCIN>>17+CARRYIN)
      | RNDSIMPLE((D-ACIN)+C)
      | RNDSIMPLE((D-ACIN)+C+CARRYIN)
      | RNDSIMPLE((D-ACIN)+CARRYIN)
      | RNDSIMPLE((D-ACIN)+P)
      | RNDSIMPLE((D-ACIN)+P+CARRYIN)
      | RNDSIMPLE((D-ACIN)+P>>17)
      | RNDSIMPLE((D-ACIN)+P>>17+CARRYIN)
      | RNDSIMPLE((D-ACIN)+PCIN)
      | RNDSIMPLE((D-ACIN)+PCIN+CARRYIN)
      | RNDSIMPLE((D-ACIN)+PCIN>>17)
      | RNDSIMPLE((D-ACIN)+PCIN>>17+CARRYIN)
      | RNDSIMPLE(A)
      | RNDSIMPLE(A*A)
      | RNDSIMPLE(A*A+C)
      | RNDSIMPLE(A*A+C+CARRYIN)
      | RNDSIMPLE(A*A+CARRYIN)
      | RNDSIMPLE(A*A+P)
      | RNDSIMPLE(A*A+P+CARRYIN)
      | RNDSIMPLE(A*A+P>>17)
      | RNDSIMPLE(A*A+P>>17+CARRYIN)
      | RNDSIMPLE(A*A+PCIN)
      | RNDSIMPLE(A*A+PCIN+CARRYIN)
      | RNDSIMPLE(A*A+PCIN>>17)
      | RNDSIMPLE(A*A+PCIN>>17+CARRYIN)
      | RNDSIMPLE(A*B)
      | RNDSIMPLE(A*B+C)
      | RNDSIMPLE(A*B+C+CARRYIN)
      | RNDSIMPLE(A*B+CARRYIN)
      | RNDSIMPLE(A*B+P)
      | RNDSIMPLE(A*B+P+CARRYIN)
      | RNDSIMPLE(A*B+P>>17)
      | RNDSIMPLE(A*B+P>>17+CARRYIN)
      | RNDSIMPLE(A*B+PCIN)
      | RNDSIMPLE(A*B+PCIN+CARRYIN)
      | RNDSIMPLE(A*B+PCIN>>17)
      | RNDSIMPLE(A*B+PCIN>>17+CARRYIN)
      | RNDSIMPLE(A*BCIN)
      | RNDSIMPLE(A*BCIN+C)
      | RNDSIMPLE(A*BCIN+C+CARRYIN)
      | RNDSIMPLE(A*BCIN+CARRYIN)
      | RNDSIMPLE(A*BCIN+P)
      | RNDSIMPLE(A*BCIN+P+CARRYIN)
      | RNDSIMPLE(A*BCIN+P>>17)
      | RNDSIMPLE(A*BCIN+P>>17+CARRYIN)
      | RNDSIMPLE(A*BCIN+PCIN)
      | RNDSIMPLE(A*BCIN+PCIN+CARRYIN)
      | RNDSIMPLE(A*BCIN+PCIN>>17)
      | RNDSIMPLE(A*BCIN+PCIN>>17+CARRYIN)
      | RNDSIMPLE(A+C)
      | RNDSIMPLE(A+C+CARRYIN)
      | RNDSIMPLE(A+CARRYIN)
      | RNDSIMPLE(A+P)
      | RNDSIMPLE(A+P+CARRYIN)
      | RNDSIMPLE(A+P>>17)
      | RNDSIMPLE(A+P>>17+CARRYIN)
      | RNDSIMPLE(A+PCIN)
      | RNDSIMPLE(A+PCIN+CARRYIN)
      | RNDSIMPLE(A+PCIN>>17)
      | RNDSIMPLE(A+PCIN>>17+CARRYIN)
      | RNDSIMPLE(ACIN)
      | RNDSIMPLE(ACIN*ACIN)
      | RNDSIMPLE(ACIN*ACIN+C)
      | RNDSIMPLE(ACIN*ACIN+C+CARRYIN)
      | RNDSIMPLE(ACIN*ACIN+CARRYIN)
      | RNDSIMPLE(ACIN*ACIN+P)
      | RNDSIMPLE(ACIN*ACIN+P+CARRYIN)
      | RNDSIMPLE(ACIN*ACIN+P>>17)
      | RNDSIMPLE(ACIN*ACIN+P>>17+CARRYIN)
      | RNDSIMPLE(ACIN*ACIN+PCIN)
      | RNDSIMPLE(ACIN*ACIN+PCIN+CARRYIN)
      | RNDSIMPLE(ACIN*ACIN+PCIN>>17)
      | RNDSIMPLE(ACIN*ACIN+PCIN>>17+CARRYIN)
      | RNDSIMPLE(ACIN*B)
      | RNDSIMPLE(ACIN*B+C)
      | RNDSIMPLE(ACIN*B+C+CARRYIN)
      | RNDSIMPLE(ACIN*B+CARRYIN)